simulink model "AcasXuClosedLoop_all_2017b"  (Simulink 9.0)
settings: Solver=VariableStepAuto  StartTime=0.0  StopTime=20  RelTol=1e-3
libraries: neural x14040, simulink x2
(part 12 of 534)

== system AcasXuClosedLoop_all_2017b/networks/Networks_m_2/AcasXu_4_2/Layer 3 ==
  "a{2} " : Inport  PortDimensions=50
      mask Display: e = 0.06;c1 = [120 230 180]/255;c2 = ([120 230 180]+0)/(256+128);c3 = ([120 230 180]+128)/(256+128);patch([0 1 1 0],[0 0 1 1],c1);patch([0 1 1 1-e 1-e 0+e],[0 0 1 1-e 0+e 0+e],c2);patch([0 0 1 1-e 0+e 0+e],[0 1 1 1-e 1-e 0+e],c3);plot([0 1 1 0 0],[0 0 1 1 0]);
  Delays 1 : Subsystem
      mask Display: e = 0.06;c1 = [120 150 230]/255;c2 = ([120 150 230]+0)/383;c3 = ([120 150 230]+128)/383;patch([0 1 1 0],[0 0 1 1],c1);patch([0 1 1 1-e 1-e 0+e],[0 0 1 1-e 0+e 0+e],c2);patch([0 0 1 1-e 0+e 0+e],[0 1 1 1-e 1-e 0+e],c3);plot([0 1 1 0 0],[0 0 1 1 0]);disp('0');
  "LW{3,2}" : Subsystem
      mask Display: e = 0.06;c1 = [120 150 230]/255;c2 = ([120 150 230]+0)/383;c3 = ([120 150 230]+128)/383;patch([0 1 1 0],[0 0 1 1],c1);patch([0 1 1 1-e 1-e 0+e],[0 0 1 1-e 0+e 0+e],c2);patch([0 0 1 1-e 0+e 0+e],[0 1 1 1-e 1-e 0+e],c3);plot([0 1 1 0 0],[0 0 1 1 0]);disp('W');
  "b{3}" : Constant  Value=[-1.2093100000000001070787902790470980107784271240234375;-0.3852510000000000101039177025086246430873870849609375;-1.1825900000000000300559577226522378623485565185546875;0.373638999999999998902211473250645212829113006591796875;-1.0167500000000000426325641456060111522674560546875;-0.17659700000000000397193389289896003901958465576171875;-0.289461000000000023835156071072560735046863555908203125;-1.035530000000000061533000916824676096439361572265625;0.197462999999999999634070491083548404276371002197265625;-0.62217000000000000081712414612411521375179290771484375;-1.1047800000000000952837808654294349253177642822265625;1.0904000000000000358824081558850593864917755126953125;-0.0993316999999999949988449543525348417460918426513671875;0.144187000000000009602985073797754012048244476318359375;-0.01483650000000000072908346027134030009619891643524169921875;0.123421000000000002927436071331612765789031982421875;-1.0655699999999999061373046060907654464244842529296875;-0.013062600000000000599609251139554544351994991302490234375;-0.5942600000000000104449782156734727323055267333984375;-0.4652439999999999908908421275555156171321868896484375;-0.395979000000000025405455517102382145822048187255859375;-0.0127361000000000001985966946449480019509792327880859375;-1.1045100000000001028155338644864968955516815185546875;-0.1830179999999999862492217062026611529290676116943359375;0.90672699999999994968646888082730583846569061279296875;-0.57416999999999995818456000051810406148433685302734375;-0.6274889999999999634638925272156484425067901611328125;0.69388600000000000278532752417959272861480712890625;-0.285387000000000001787014980436651967465877532958984375;1.4988099999999999756283841634285636246204376220703125;0.04194029999999999980264675514263217337429523468017578125;-0.39841300000000001713118535917601548135280609130859375;-0.486005999999999993566035527692292816936969757080078125;-1.3225100000000000743938244340824894607067108154296875;0.375159000000000020236257114447653293609619140625;-0.42299100000000000587618842473602853715419769287109375;0.38622800000000001574562702444382011890411376953125;-1.039639999999999897539737503393553197383880615234375;-1.2919899999999999717914533903240226209163665771484375;0.93974400000000002375344365646014921367168426513671875;-0.371230000000000004423128530106623657047748565673828125;-0.34603200000000000624567064733128063380718231201171875;-0.36628899999999997572075471907737664878368377685546875;-1.4668200000000000127187149701057933270931243896484375;0.79338900000000001089262013920233584940433502197265625;-0.47510799999999997478283830787404440343379974365234375;0.552489000000000007872813512221910059452056884765625;2.261649999999999938182781988871283829212188720703125;1.162819999999999964757080306299030780792236328125;0.258203000000000015834444866413832642138004302978515625]
      mask Display: e = 0.06;c1 = [120 150 230]/255;c2 = ([120 150 230]+0)/383;c3 = ([120 150 230]+128)/383;patch([0 1 1 0],[0 0 1 1],c1);patch([0 1 1 1-e 1-e 0+e],[0 0 1 1-e 0+e 0+e],c2);patch([0 0 1 1-e 0+e 0+e],[0 1 1 1-e 1-e 0+e],c3);plot([0 1 1 0 0],[0 0 1 1 0]);disp('b');
  netsum : Sum  Inputs=2  SaturateOnIntegerOverflow=on  InputSameDT=on  OutDataTypeStr=Inherit: Same as first input
      mask Display: e = 0.06;c1 = [120 150 230]/255;c2 = ([120 150 230]+0)/383;c3 = ([120 150 230]+128)/383;patch([0 1 1 0],[0 0 1 1],c1);patch([0 1 1 1-e 1-e 0+e],[0 0 1 1-e 0+e 0+e],c2);patch([0 0 1 1-e 0+e 0+e],[0 1 1 1-e 1-e 0+e],c3);plot([0 1 1 0 0],[0 0 1 1 0]);disp('+');
  poslin : neural/Transfer Functions/poslin
      settings: SourceProductName=Deep Learning Toolbox  SourceProductBaseCode=NN
  "a{3}" : Outport  InitialOutput=[0;0;0;0;0;0;0;0;0;0;0;0;0;0;0;0;0;0;0;0;0;0;0;0;0;0;0;0;0;0;0;0;0;0;0;0;0;0;0;0;0;0;0;0;0;0;0;0;0;0]
      mask Display: e = 0.06;c1 = [120 230 180]/255;c2 = ([120 230 180]+0)/(256+128);c3 = ([120 230 180]+128)/(256+128);patch([0 1 1 0],[0 0 1 1],c1);patch([0 1 1 1-e 1-e 0+e],[0 0 1 1-e 0+e 0+e],c2);patch([0 0 1 1-e 0+e 0+e],[0 1 1 1-e 1-e 0+e],c3);plot([0 1 1 0 0],[0 0 1 1 0]);
  wires:
  poslin/1 -> "a{3}"/1
  netsum/1 -> poslin/1
  "b{3}"/1 -> netsum/2
  "LW{3,2}"/1("lz{3,2}") -> netsum/1
  Delays 1/1("pd{3,2}") -> "LW{3,2}"/1("ad{3,2}")
  "a{2} "/1 -> Delays 1/1("p{3}")

== system AcasXuClosedLoop_all_2017b/networks/Networks_m_2/AcasXu_4_2/Layer 4 ==
  "a{3} " : Inport  PortDimensions=50
      mask Display: e = 0.06;c1 = [120 230 180]/255;c2 = ([120 230 180]+0)/(256+128);c3 = ([120 230 180]+128)/(256+128);patch([0 1 1 0],[0 0 1 1],c1);patch([0 1 1 1-e 1-e 0+e],[0 0 1 1-e 0+e 0+e],c2);patch([0 0 1 1-e 0+e 0+e],[0 1 1 1-e 1-e 0+e],c3);plot([0 1 1 0 0],[0 0 1 1 0]);
  Delays 1 : Subsystem
      mask Display: e = 0.06;c1 = [120 150 230]/255;c2 = ([120 150 230]+0)/383;c3 = ([120 150 230]+128)/383;patch([0 1 1 0],[0 0 1 1],c1);patch([0 1 1 1-e 1-e 0+e],[0 0 1 1-e 0+e 0+e],c2);patch([0 0 1 1-e 0+e 0+e],[0 1 1 1-e 1-e 0+e],c3);plot([0 1 1 0 0],[0 0 1 1 0]);disp('0');
  "LW{4,3}" : Subsystem
      mask Display: e = 0.06;c1 = [120 150 230]/255;c2 = ([120 150 230]+0)/383;c3 = ([120 150 230]+128)/383;patch([0 1 1 0],[0 0 1 1],c1);patch([0 1 1 1-e 1-e 0+e],[0 0 1 1-e 0+e 0+e],c2);patch([0 0 1 1-e 0+e 0+e],[0 1 1 1-e 1-e 0+e],c3);plot([0 1 1 0 0],[0 0 1 1 0]);disp('W');
  "b{4}" : Constant  Value=[-0.48296499999999997765343096034484915435314178466796875;0.75015200000000004099121042600017972290515899658203125;-1.2147600000000000619593265582807362079620361328125;0.591026999999999969048758430290035903453826904296875;0.382948000000000010611955758577096275985240936279296875;0.6420369999999999688355956095620058476924896240234375;0.696061999999999958532725941040553152561187744140625;1.0299799999999998956212721168412826955318450927734375;-0.66000800000000003908695589416311122477054595947265625;-0.6410000000000000142108547152020037174224853515625;-0.1299849999999999894395585897655109874904155731201171875;0.30073600000000000331823457599966786801815032958984375;0.55291199999999995906563299286062829196453094482421875;0.26601200000000002621192152219009585678577423095703125;0.653343000000000007077005648170597851276397705078125;0.307491000000000014313883411887218244373798370361328125;-0.1523029999999999939408468208057456649839878082275390625;0.6957649999999999668176542400033213198184967041015625;0.11466300000000000103295150211124564521014690399169921875;0.54934000000000005048406137575511820614337921142578125;0.0273350000000000016575629757653587148524820804595947265625;-1.7362500000000000710542735760100185871124267578125;0.40176800000000001400479732183157466351985931396484375;-1.5132499999999999840127884453977458178997039794921875;-0.9088220000000000187156956599210388958454132080078125;-0.68895300000000003759481614906690083444118499755859375;-1.105890000000000039648284655413590371608734130859375;0.454156999999999977379872007077210582792758941650390625;1.407739999999999991331378623726777732372283935546875;0.42157400000000000428457269663340412080287933349609375;-0.441346999999999989316989967846893705427646636962890625;0.128890000000000004565237077258643694221973419189453125;-0.349038000000000014910739309925702400505542755126953125;-0.159445000000000003392841563254478387534618377685546875;0.152144000000000001460165321987005881965160369873046875;0.97558000000000000273558953267638571560382843017578125;0.01782229999999999925375249176795477978885173797607421875;0.5652470000000000549533751836861483752727508544921875;-0.01870410000000000128039800983970053493976593017578125;-0.370516999999999985249132805620320141315460205078125;-0.2447870000000000045847770024920464493334293365478515625;0.80906900000000003814193405560217797756195068359375;-0.448537000000000019017676322619081474840641021728515625;0.07267600000000000448618919790533254854381084442138671875;0.1906960000000000043929304638368193991482257843017578125;-0.0632713000000000025391244662387180142104625701904296875;0.6713750000000000550670620214077644050121307373046875;-2.451470000000000037942982089589349925518035888671875;0.0585482999999999975671016727574169635772705078125;-0.60775900000000004919087359667173586785793304443359375]
      mask Display: e = 0.06;c1 = [120 150 230]/255;c2 = ([120 150 230]+0)/383;c3 = ([120 150 230]+128)/383;patch([0 1 1 0],[0 0 1 1],c1);patch([0 1 1 1-e 1-e 0+e],[0 0 1 1-e 0+e 0+e],c2);patch([0 0 1 1-e 0+e 0+e],[0 1 1 1-e 1-e 0+e],c3);plot([0 1 1 0 0],[0 0 1 1 0]);disp('b');
  netsum : Sum  Inputs=2  SaturateOnIntegerOverflow=on  InputSameDT=on  OutDataTypeStr=Inherit: Same as first input
      mask Display: e = 0.06;c1 = [120 150 230]/255;c2 = ([120 150 230]+0)/383;c3 = ([120 150 230]+128)/383;patch([0 1 1 0],[0 0 1 1],c1);patch([0 1 1 1-e 1-e 0+e],[0 0 1 1-e 0+e 0+e],c2);patch([0 0 1 1-e 0+e 0+e],[0 1 1 1-e 1-e 0+e],c3);plot([0 1 1 0 0],[0 0 1 1 0]);disp('+');
  poslin : neural/Transfer Functions/poslin
      settings: SourceProductName=Deep Learning Toolbox  SourceProductBaseCode=NN
  "a{4}" : Outport  InitialOutput=[0;0;0;0;0;0;0;0;0;0;0;0;0;0;0;0;0;0;0;0;0;0;0;0;0;0;0;0;0;0;0;0;0;0;0;0;0;0;0;0;0;0;0;0;0;0;0;0;0;0]
      mask Display: e = 0.06;c1 = [120 230 180]/255;c2 = ([120 230 180]+0)/(256+128);c3 = ([120 230 180]+128)/(256+128);patch([0 1 1 0],[0 0 1 1],c1);patch([0 1 1 1-e 1-e 0+e],[0 0 1 1-e 0+e 0+e],c2);patch([0 0 1 1-e 0+e 0+e],[0 1 1 1-e 1-e 0+e],c3);plot([0 1 1 0 0],[0 0 1 1 0]);
  wires:
  poslin/1 -> "a{4}"/1
  netsum/1 -> poslin/1
  "b{4}"/1 -> netsum/2
  "LW{4,3}"/1("lz{4,3}") -> netsum/1
  Delays 1/1("pd{4,3}") -> "LW{4,3}"/1("ad{4,3}")
  "a{3} "/1 -> Delays 1/1("p{4}")

== system AcasXuClosedLoop_all_2017b/networks/Networks_m_2/AcasXu_4_2/Layer 5 ==
  "a{4} " : Inport  PortDimensions=50
      mask Display: e = 0.06;c1 = [120 230 180]/255;c2 = ([120 230 180]+0)/(256+128);c3 = ([120 230 180]+128)/(256+128);patch([0 1 1 0],[0 0 1 1],c1);patch([0 1 1 1-e 1-e 0+e],[0 0 1 1-e 0+e 0+e],c2);patch([0 0 1 1-e 0+e 0+e],[0 1 1 1-e 1-e 0+e],c3);plot([0 1 1 0 0],[0 0 1 1 0]);
  Delays 1 : Subsystem
      mask Display: e = 0.06;c1 = [120 150 230]/255;c2 = ([120 150 230]+0)/383;c3 = ([120 150 230]+128)/383;patch([0 1 1 0],[0 0 1 1],c1);patch([0 1 1 1-e 1-e 0+e],[0 0 1 1-e 0+e 0+e],c2);patch([0 0 1 1-e 0+e 0+e],[0 1 1 1-e 1-e 0+e],c3);plot([0 1 1 0 0],[0 0 1 1 0]);disp('0');
  "LW{5,4}" : Subsystem
      mask Display: e = 0.06;c1 = [120 150 230]/255;c2 = ([120 150 230]+0)/383;c3 = ([120 150 230]+128)/383;patch([0 1 1 0],[0 0 1 1],c1);patch([0 1 1 1-e 1-e 0+e],[0 0 1 1-e 0+e 0+e],c2);patch([0 0 1 1-e 0+e 0+e],[0 1 1 1-e 1-e 0+e],c3);plot([0 1 1 0 0],[0 0 1 1 0]);disp('W');
  "b{5}" : Constant  Value=[1.667030000000000011795009413617663085460662841796875;-0.16405300000000000437694325228221714496612548828125;-0.036280899999999997873612045395930181257426738739013671875;-1.4923200000000000908784159037168137729167938232421875;-0.01533149999999999964217511916331204702146351337432861328125;0.89175999999999999712230192017159424722194671630859375;-1.570840000000000014068746168049983680248260498046875;-1.1088999999999999968025576890795491635799407958984375;0.75545799999999996288124748389236629009246826171875;0.43142000000000002568611989772762171924114227294921875;0.48276499999999999968025576890795491635799407958984375;1.13823000000000007503331289626657962799072265625;-1.658139999999999947277729006600566208362579345703125;-0.03238660000000000149800172266623121686279773712158203125;0.79739400000000004720135393654345534741878509521484375;-0.489690000000000014157564010019996203482151031494140625;-0.54653600000000002179234570576227270066738128662109375;0.61249500000000001165290086646564304828643798828125;0.65238899999999999668176542400033213198184967041015625;-1.121070000000000010942358130705542862415313720703125;0.64722699999999999675992512493394315242767333984375;0.5347910000000000163566937771975062787532806396484375;0.5378220000000000222684093387215398252010345458984375;-0.30079800000000000981259518084698356688022613525390625;-0.7375469999999999526352212342317216098308563232421875;-0.05225459999999999827213770231537637300789356231689453125;-0.01783490000000000075264239285388612188398838043212890625;1.2560599999999999543121020906255580484867095947265625;0.10386099999999999499156189131099381484091281890869140625;-0.34737099999999998534150336126913316547870635986328125;0.484240999999999977010389784481958486139774322509765625;0.2878249999999999975131004248396493494510650634765625;-0.6927529999999999521520521739148534834384918212890625;0.764796000000000031349145501735620200634002685546875;-0.033029799999999998050270733074285089969635009765625;-1.329590000000000049595882956054992973804473876953125;0.326909999999999978381737264498951844871044158935546875;0.1570979999999999876525436093288590200245380401611328125;0.4335620000000000029416469260468147695064544677734375;-0.10705199999999999438404785223610815592110157012939453125;-0.395469000000000014960477301428909413516521453857421875;-0.5508070000000000465689709017169661819934844970703125;0.363134000000000012331469179116538725793361663818359375;0.1310679999999999900683889109131996519863605499267578125;0.48874099999999998100719267313252203166484832763671875;-0.72436500000000003662847802843316458165645599365234375;0.53715699999999999558752961092977784574031829833984375;1.0689699999999999757704927105805836617946624755859375;-0.86060800000000003961986294598318636417388916015625;-0.032118599999999997152144715073518455028533935546875]
      mask Display: e = 0.06;c1 = [120 150 230]/255;c2 = ([120 150 230]+0)/383;c3 = ([120 150 230]+128)/383;patch([0 1 1 0],[0 0 1 1],c1);patch([0 1 1 1-e 1-e 0+e],[0 0 1 1-e 0+e 0+e],c2);patch([0 0 1 1-e 0+e 0+e],[0 1 1 1-e 1-e 0+e],c3);plot([0 1 1 0 0],[0 0 1 1 0]);disp('b');
  netsum : Sum  Inputs=2  SaturateOnIntegerOverflow=on  InputSameDT=on  OutDataTypeStr=Inherit: Same as first input
      mask Display: e = 0.06;c1 = [120 150 230]/255;c2 = ([120 150 230]+0)/383;c3 = ([120 150 230]+128)/383;patch([0 1 1 0],[0 0 1 1],c1);patch([0 1 1 1-e 1-e 0+e],[0 0 1 1-e 0+e 0+e],c2);patch([0 0 1 1-e 0+e 0+e],[0 1 1 1-e 1-e 0+e],c3);plot([0 1 1 0 0],[0 0 1 1 0]);disp('+');
  poslin : neural/Transfer Functions/poslin
      settings: SourceProductName=Deep Learning Toolbox  SourceProductBaseCode=NN
  "a{5}" : Outport  InitialOutput=[0;0;0;0;0;0;0;0;0;0;0;0;0;0;0;0;0;0;0;0;0;0;0;0;0;0;0;0;0;0;0;0;0;0;0;0;0;0;0;0;0;0;0;0;0;0;0;0;0;0]
      mask Display: e = 0.06;c1 = [120 230 180]/255;c2 = ([120 230 180]+0)/(256+128);c3 = ([120 230 180]+128)/(256+128);patch([0 1 1 0],[0 0 1 1],c1);patch([0 1 1 1-e 1-e 0+e],[0 0 1 1-e 0+e 0+e],c2);patch([0 0 1 1-e 0+e 0+e],[0 1 1 1-e 1-e 0+e],c3);plot([0 1 1 0 0],[0 0 1 1 0]);
  wires:
  poslin/1 -> "a{5}"/1
  netsum/1 -> poslin/1
  "b{5}"/1 -> netsum/2
  "LW{5,4}"/1("lz{5,4}") -> netsum/1
  Delays 1/1("pd{5,4}") -> "LW{5,4}"/1("ad{5,4}")
  "a{4} "/1 -> Delays 1/1("p{5}")

== system AcasXuClosedLoop_all_2017b/networks/Networks_m_2/AcasXu_4_2/Layer 6 ==
  "a{5} " : Inport  PortDimensions=50
      mask Display: e = 0.06;c1 = [120 230 180]/255;c2 = ([120 230 180]+0)/(256+128);c3 = ([120 230 180]+128)/(256+128);patch([0 1 1 0],[0 0 1 1],c1);patch([0 1 1 1-e 1-e 0+e],[0 0 1 1-e 0+e 0+e],c2);patch([0 0 1 1-e 0+e 0+e],[0 1 1 1-e 1-e 0+e],c3);plot([0 1 1 0 0],[0 0 1 1 0]);
  Delays 1 : Subsystem
      mask Display: e = 0.06;c1 = [120 150 230]/255;c2 = ([120 150 230]+0)/383;c3 = ([120 150 230]+128)/383;patch([0 1 1 0],[0 0 1 1],c1);patch([0 1 1 1-e 1-e 0+e],[0 0 1 1-e 0+e 0+e],c2);patch([0 0 1 1-e 0+e 0+e],[0 1 1 1-e 1-e 0+e],c3);plot([0 1 1 0 0],[0 0 1 1 0]);disp('0');
  "LW{6,5}" : Subsystem
      mask Display: e = 0.06;c1 = [120 150 230]/255;c2 = ([120 150 230]+0)/383;c3 = ([120 150 230]+128)/383;patch([0 1 1 0],[0 0 1 1],c1);patch([0 1 1 1-e 1-e 0+e],[0 0 1 1-e 0+e 0+e],c2);patch([0 0 1 1-e 0+e 0+e],[0 1 1 1-e 1-e 0+e],c3);plot([0 1 1 0 0],[0 0 1 1 0]);disp('W');
  "b{6}" : Constant  Value=[0.271396000000000026108892825504881329834461212158203125;-0.14563599999999998768629438927746377885341644287109375;0.009515980000000000271764832859844318591058254241943359375;-0.112346000000000001417532757841399870812892913818359375;-0.6355239999999999778168557895696721971035003662109375;-0.045955000000000002791100683907643542625010013580322265625;0.2134729999999999960902385964800487272441387176513671875;-1.18484999999999995878852132591418921947479248046875;0.2363860000000000127773347458060015924274921417236328125;-0.153476000000000001310951347477384842932224273681640625;-0.64991600000000004921929530610213987529277801513671875;-0.359387999999999985245580091941519640386104583740234375;-0.63418200000000002347633198951371014118194580078125;-0.206660000000000010356160373703460209071636199951171875;0.056250399999999999012256779451490729115903377532958984375;0.047399400000000001254019110774606815539300441741943359375;0.4504230000000000178062009581481106579303741455078125;-0.58878099999999999880628820392303168773651123046875;0.08031670000000000475548489475841051898896694183349609375;-2.12455000000000016058265828178264200687408447265625;-0.633866999999999958248508846736513078212738037109375;0.248182999999999986950882657765760086476802825927734375;-0.10464700000000000390532051142145064659416675567626953125;-1.4727799999999999780442294650129042565822601318359375;0.14918799999999998728839045725180767476558685302734375;0.0757379999999999997672972540385671891272068023681640625;0.471032999999999979490183932284708134829998016357421875;-0.69371499999999997054800360274384729564189910888671875;-0.32937899999999997735500301132560707628726959228515625;-1.407859999999999889297441768576391041278839111328125;0.340706000000000008842704346534446813166141510009765625;-0.0423916000000000015024426147647318430244922637939453125;-0.0020437699999999998749788954199857471394352614879608154296875;-0.06183249999999999857447363638129900209605693817138671875;0.07975949999999999706634667973048635758459568023681640625;0.2066989999999999938484762651569326408207416534423828125;-0.3627059999999999728714783486793749034404754638671875;-0.0870270999999999961271868187395739369094371795654296875;-0.2309420000000000083861806388085824437439441680908203125;-0.2197610000000000118891563261058763600885868072509765625;-0.79645799999999999929656269159750081598758697509765625;0.1211170000000000024353852268177433870732784271240234375;-0.07685419999999999751683077420238987542688846588134765625;-0.06845890000000000308322256614701473154127597808837890625;0.135371999999999992336796594827319495379924774169921875;0.2409030000000000060200733287274488247931003570556640625;0.042481499999999998429256464760328526608645915985107421875;0.275341000000000002412292587905540131032466888427734375;0.145892999999999994908961298278882168233394622802734375;-0.029134000000000000174527059471074608154594898223876953125]
      mask Display: e = 0.06;c1 = [120 150 230]/255;c2 = ([120 150 230]+0)/383;c3 = ([120 150 230]+128)/383;patch([0 1 1 0],[0 0 1 1],c1);patch([0 1 1 1-e 1-e 0+e],[0 0 1 1-e 0+e 0+e],c2);patch([0 0 1 1-e 0+e 0+e],[0 1 1 1-e 1-e 0+e],c3);plot([0 1 1 0 0],[0 0 1 1 0]);disp('b');
  netsum : Sum  Inputs=2  SaturateOnIntegerOverflow=on  InputSameDT=on  OutDataTypeStr=Inherit: Same as first input
      mask Display: e = 0.06;c1 = [120 150 230]/255;c2 = ([120 150 230]+0)/383;c3 = ([120 150 230]+128)/383;patch([0 1 1 0],[0 0 1 1],c1);patch([0 1 1 1-e 1-e 0+e],[0 0 1 1-e 0+e 0+e],c2);patch([0 0 1 1-e 0+e 0+e],[0 1 1 1-e 1-e 0+e],c3);plot([0 1 1 0 0],[0 0 1 1 0]);disp('+');
  poslin : neural/Transfer Functions/poslin
      settings: SourceProductName=Deep Learning Toolbox  SourceProductBaseCode=NN
  "a{6}" : Outport  InitialOutput=[0;0;0;0;0;0;0;0;0;0;0;0;0;0;0;0;0;0;0;0;0;0;0;0;0;0;0;0;0;0;0;0;0;0;0;0;0;0;0;0;0;0;0;0;0;0;0;0;0;0]
      mask Display: e = 0.06;c1 = [120 230 180]/255;c2 = ([120 230 180]+0)/(256+128);c3 = ([120 230 180]+128)/(256+128);patch([0 1 1 0],[0 0 1 1],c1);patch([0 1 1 1-e 1-e 0+e],[0 0 1 1-e 0+e 0+e],c2);patch([0 0 1 1-e 0+e 0+e],[0 1 1 1-e 1-e 0+e],c3);plot([0 1 1 0 0],[0 0 1 1 0]);
  wires:
  poslin/1 -> "a{6}"/1
  netsum/1 -> poslin/1
  "b{6}"/1 -> netsum/2
  "LW{6,5}"/1("lz{6,5}") -> netsum/1
  Delays 1/1("pd{6,5}") -> "LW{6,5}"/1("ad{6,5}")
  "a{5} "/1 -> Delays 1/1("p{6}")

== system AcasXuClosedLoop_all_2017b/networks/Networks_m_2/AcasXu_4_2/Layer 7 ==
  "a{6} " : Inport  PortDimensions=50
      mask Display: e = 0.06;c1 = [120 230 180]/255;c2 = ([120 230 180]+0)/(256+128);c3 = ([120 230 180]+128)/(256+128);patch([0 1 1 0],[0 0 1 1],c1);patch([0 1 1 1-e 1-e 0+e],[0 0 1 1-e 0+e 0+e],c2);patch([0 0 1 1-e 0+e 0+e],[0 1 1 1-e 1-e 0+e],c3);plot([0 1 1 0 0],[0 0 1 1 0]);
  Delays 1 : Subsystem
      mask Display: e = 0.06;c1 = [120 150 230]/255;c2 = ([120 150 230]+0)/383;c3 = ([120 150 230]+128)/383;patch([0 1 1 0],[0 0 1 1],c1);patch([0 1 1 1-e 1-e 0+e],[0 0 1 1-e 0+e 0+e],c2);patch([0 0 1 1-e 0+e 0+e],[0 1 1 1-e 1-e 0+e],c3);plot([0 1 1 0 0],[0 0 1 1 0]);disp('0');
  "LW{7,6}" : Subsystem
      mask Display: e = 0.06;c1 = [120 150 230]/255;c2 = ([120 150 230]+0)/383;c3 = ([120 150 230]+128)/383;patch([0 1 1 0],[0 0 1 1],c1);patch([0 1 1 1-e 1-e 0+e],[0 0 1 1-e 0+e 0+e],c2);patch([0 0 1 1-e 0+e 0+e],[0 1 1 1-e 1-e 0+e],c3);plot([0 1 1 0 0],[0 0 1 1 0]);disp('W');
  "b{7}" : Constant  Value=[0.01468369999999999918338655646721235825680196285247802734375;-0.00470128000000000038915981548370837117545306682586669921875;0.035427699999999999469491029913115198723971843719482421875;-0.000654970999999999960346219740614515103516168892383575439453125;0.03616529999999999744897394293730030767619609832763671875]
      mask Display: e = 0.06;c1 = [120 150 230]/255;c2 = ([120 150 230]+0)/383;c3 = ([120 150 230]+128)/383;patch([0 1 1 0],[0 0 1 1],c1);patch([0 1 1 1-e 1-e 0+e],[0 0 1 1-e 0+e 0+e],c2);patch([0 0 1 1-e 0+e 0+e],[0 1 1 1-e 1-e 0+e],c3);plot([0 1 1 0 0],[0 0 1 1 0]);disp('b');
  netsum : Sum  Inputs=2  SaturateOnIntegerOverflow=on  InputSameDT=on  OutDataTypeStr=Inherit: Same as first input
      mask Display: e = 0.06;c1 = [120 150 230]/255;c2 = ([120 150 230]+0)/383;c3 = ([120 150 230]+128)/383;patch([0 1 1 0],[0 0 1 1],c1);patch([0 1 1 1-e 1-e 0+e],[0 0 1 1-e 0+e 0+e],c2);patch([0 0 1 1-e 0+e 0+e],[0 1 1 1-e 1-e 0+e],c3);plot([0 1 1 0 0],[0 0 1 1 0]);disp('+');
  purelin : neural/Transfer Functions/purelin
      settings: SourceProductName=Deep Learning Toolbox
  "a{7}" : Outport  InitialOutput=[0;0;0;0;0]
      mask Display: e = 0.06;c1 = [120 230 180]/255;c2 = ([120 230 180]+0)/(256+128);c3 = ([120 230 180]+128)/(256+128);patch([0 1 1 0],[0 0 1 1],c1);patch([0 1 1 1-e 1-e 0+e],[0 0 1 1-e 0+e 0+e],c2);patch([0 0 1 1-e 0+e 0+e],[0 1 1 1-e 1-e 0+e],c3);plot([0 1 1 0 0],[0 0 1 1 0]);
  wires:
  purelin/1 -> "a{7}"/1
  netsum/1 -> purelin/1
  "b{7}"/1 -> netsum/2
  "LW{7,6}"/1("lz{7,6}") -> netsum/1
  Delays 1/1("pd{7,6}") -> "LW{7,6}"/1("ad{7,6}")
  "a{6} "/1 -> Delays 1/1("p{7}")

== system AcasXuClosedLoop_all_2017b/networks/Networks_m_2/AcasXu_4_2/Process Input 1 ==
  x : Inport  PortDimensions=5
      mask Display: e = 0.06;c1 = [120 230 180]/255;c2 = ([120 230 180]+0)/(256+128);c3 = ([120 230 180]+128)/(256+128);patch([0 1 1 0],[0 0 1 1],c1);patch([0 1 1 1-e 1-e 0+e],[0 0 1 1-e 0+e 0+e],c2);patch([0 0 1 1-e 0+e 0+e],[0 1 1 1-e 1-e 0+e],c3);plot([0 1 1 0 0],[0 0 1 1 0]);
  p : Outport  PortDimensions=5
      mask Display: e = 0.06;c1 = [120 230 180]/255;c2 = ([120 230 180]+0)/(256+128);c3 = ([120 230 180]+128)/(256+128);patch([0 1 1 0],[0 0 1 1],c1);patch([0 1 1 1-e 1-e 0+e],[0 0 1 1-e 0+e 0+e],c2);patch([0 0 1 1-e 0+e 0+e],[0 1 1 1-e 1-e 0+e],c3);plot([0 1 1 0 0],[0 0 1 1 0]);
  wires:
  x/1 -> p/1

== system AcasXuClosedLoop_all_2017b/networks/Networks_m_2/AcasXu_4_2/Process Output 1 ==
  a : Inport  PortDimensions=5
      mask Display: e = 0.06;c1 = [120 230 180]/255;c2 = ([120 230 180]+0)/(256+128);c3 = ([120 230 180]+128)/(256+128);patch([0 1 1 0],[0 0 1 1],c1);patch([0 1 1 1-e 1-e 0+e],[0 0 1 1-e 0+e 0+e],c2);patch([0 0 1 1-e 0+e 0+e],[0 1 1 1-e 1-e 0+e],c3);plot([0 1 1 0 0],[0 0 1 1 0]);
  y : Outport  PortDimensions=5
      mask Display: e = 0.06;c1 = [120 230 180]/255;c2 = ([120 230 180]+0)/(256+128);c3 = ([120 230 180]+128)/(256+128);patch([0 1 1 0],[0 0 1 1],c1);patch([0 1 1 1-e 1-e 0+e],[0 0 1 1-e 0+e 0+e],c2);patch([0 0 1 1-e 0+e 0+e],[0 1 1 1-e 1-e 0+e],c3);plot([0 1 1 0 0],[0 0 1 1 0]);
  wires:
  a/1 -> y/1

== system AcasXuClosedLoop_all_2017b/networks/Networks_m_2/AcasXu_5_2/Layer 1 ==
  "p{1}" : Inport  PortDimensions=5
      mask Display: e = 0.06;c1 = [120 230 180]/255;c2 = ([120 230 180]+0)/(256+128);c3 = ([120 230 180]+128)/(256+128);patch([0 1 1 0],[0 0 1 1],c1);patch([0 1 1 1-e 1-e 0+e],[0 0 1 1-e 0+e 0+e],c2);patch([0 0 1 1-e 0+e 0+e],[0 1 1 1-e 1-e 0+e],c3);plot([0 1 1 0 0],[0 0 1 1 0]);
  Delays 1 : Subsystem
      mask Display: e = 0.06;c1 = [120 150 230]/255;c2 = ([120 150 230]+0)/383;c3 = ([120 150 230]+128)/383;patch([0 1 1 0],[0 0 1 1],c1);patch([0 1 1 1-e 1-e 0+e],[0 0 1 1-e 0+e 0+e],c2);patch([0 0 1 1-e 0+e 0+e],[0 1 1 1-e 1-e 0+e],c3);plot([0 1 1 0 0],[0 0 1 1 0]);disp('0');
  "IW{1,1}" : Subsystem
      mask Display: e = 0.06;c1 = [120 150 230]/255;c2 = ([120 150 230]+0)/383;c3 = ([120 150 230]+128)/383;patch([0 1 1 0],[0 0 1 1],c1);patch([0 1 1 1-e 1-e 0+e],[0 0 1 1-e 0+e 0+e],c2);patch([0 0 1 1-e 0+e 0+e],[0 1 1 1-e 1-e 0+e],c3);plot([0 1 1 0 0],[0 0 1 1 0]);disp('W');
  "b{1}" : Constant  Value=[-0.5225699999999999789679350215010344982147216796875;0.08098919999999999730366795347435981966555118560791015625;-0.388828000000000006952660669412580318748950958251953125;-0.423870000000000024531487952117458917200565338134765625;0.363358000000000014306777984529617242515087127685546875;0.113070000000000003836930773104541003704071044921875;-0.007512839999999999796020944131669239141047000885009765625;-0.44695099999999998718891447424539364874362945556640625;-0.024655400000000000926281273905260604806244373321533203125;0.00349412999999999980549336697777107474394142627716064453125;0.1640500000000000013766765505351941101253032684326171875;0.151363999999999998546940105370595119893550872802734375;-0.7931340000000000056701310313655994832515716552734375;-0.257234000000000018193446749137365259230136871337890625;-0.044330799999999996596766749235030147247016429901123046875;-0.1867379999999999873327993782368139363825321197509765625;0.047071899999999999852917653697659261524677276611328125;-0.11033800000000000551825252159687806852161884307861328125;-0.526672000000000029018565328442491590976715087890625;-0.294516999999999973258724139668629504740238189697265625;-0.01289189999999999954038987226567769539542496204376220703125;0.13947100000000001163158458439284004271030426025390625;-0.7452140000000000430446789323468692600727081298828125;-0.3850379999999999913740111878723837435245513916015625;-0.1279039999999999899227276500823791138827800750732421875;-0.1974829999999999918802728871014551259577274322509765625;0.2259859999999999924380489346731337718665599822998046875;-0.41572799999999998643573917433968745172023773193359375;0.0689332000000000000294875235340441577136516571044921875;-0.31816100000000002712141622396302409470081329345703125;0.54194399999999998129851519479416310787200927734375;-0.10822600000000000275424127949008834548294544219970703125;-0.427329999999999987636556397774256765842437744140625;-0.465955999999999981309173335830564610660076141357421875;-0.5985270000000000312212478092988021671772003173828125;-0.0249557000000000007988720795992776402272284030914306640625;-0.492057000000000022144064359963522292673587799072265625;-0.76232200000000005513101086762617342174053192138671875;0.060068000000000003335554055183820310048758983612060546875;-0.7915039999999999853486087886267341673374176025390625;0.25127500000000002611244553918368183076381683349609375;0.1326779999999999903881331420052447356283664703369140625;0.62141900000000005466205266202450729906558990478515625;-0.56192600000000003657163460957235656678676605224609375;0.093970100000000000850519654704839922487735748291015625;-0.0257846000000000012686296457786738756112754344940185546875;0.008458040000000000002700062395888380706310272216796875;-0.2342750000000000110134124042815528810024261474609375;-0.1987899999999999944844120136622223071753978729248046875;-0.0026113299999999998145250312830967232002876698970794677734375]
      mask Display: e = 0.06;c1 = [120 150 230]/255;c2 = ([120 150 230]+0)/383;c3 = ([120 150 230]+128)/383;patch([0 1 1 0],[0 0 1 1],c1);patch([0 1 1 1-e 1-e 0+e],[0 0 1 1-e 0+e 0+e],c2);patch([0 0 1 1-e 0+e 0+e],[0 1 1 1-e 1-e 0+e],c3);plot([0 1 1 0 0],[0 0 1 1 0]);disp('b');
  netsum : Sum  Inputs=2  SaturateOnIntegerOverflow=on  InputSameDT=on  OutDataTypeStr=Inherit: Same as first input
      mask Display: e = 0.06;c1 = [120 150 230]/255;c2 = ([120 150 230]+0)/383;c3 = ([120 150 230]+128)/383;patch([0 1 1 0],[0 0 1 1],c1);patch([0 1 1 1-e 1-e 0+e],[0 0 1 1-e 0+e 0+e],c2);patch([0 0 1 1-e 0+e 0+e],[0 1 1 1-e 1-e 0+e],c3);plot([0 1 1 0 0],[0 0 1 1 0]);disp('+');
  poslin : neural/Transfer Functions/poslin
      settings: SourceProductName=Deep Learning Toolbox  SourceProductBaseCode=NN
  "a{1}" : Outport  InitialOutput=[0;0;0;0;0;0;0;0;0;0;0;0;0;0;0;0;0;0;0;0;0;0;0;0;0;0;0;0;0;0;0;0;0;0;0;0;0;0;0;0;0;0;0;0;0;0;0;0;0;0]
      mask Display: e = 0.06;c1 = [120 230 180]/255;c2 = ([120 230 180]+0)/(256+128);c3 = ([120 230 180]+128)/(256+128);patch([0 1 1 0],[0 0 1 1],c1);patch([0 1 1 1-e 1-e 0+e],[0 0 1 1-e 0+e 0+e],c2);patch([0 0 1 1-e 0+e 0+e],[0 1 1 1-e 1-e 0+e],c3);plot([0 1 1 0 0],[0 0 1 1 0]);
  wires:
  poslin/1 -> "a{1}"/1
  netsum/1 -> poslin/1
  "b{1}"/1 -> netsum/2
  "IW{1,1}"/1("iz{1,1}") -> netsum/1
  Delays 1/1("pd{1,1}") -> "IW{1,1}"/1("pd{1,1}")
  "p{1}"/1 -> Delays 1/1("p{1}")

== system AcasXuClosedLoop_all_2017b/networks/Networks_m_2/AcasXu_5_2/Layer 2 ==
  "a{1} " : Inport  PortDimensions=50
      mask Display: e = 0.06;c1 = [120 230 180]/255;c2 = ([120 230 180]+0)/(256+128);c3 = ([120 230 180]+128)/(256+128);patch([0 1 1 0],[0 0 1 1],c1);patch([0 1 1 1-e 1-e 0+e],[0 0 1 1-e 0+e 0+e],c2);patch([0 0 1 1-e 0+e 0+e],[0 1 1 1-e 1-e 0+e],c3);plot([0 1 1 0 0],[0 0 1 1 0]);
  Delays 1 : Subsystem
      mask Display: e = 0.06;c1 = [120 150 230]/255;c2 = ([120 150 230]+0)/383;c3 = ([120 150 230]+128)/383;patch([0 1 1 0],[0 0 1 1],c1);patch([0 1 1 1-e 1-e 0+e],[0 0 1 1-e 0+e 0+e],c2);patch([0 0 1 1-e 0+e 0+e],[0 1 1 1-e 1-e 0+e],c3);plot([0 1 1 0 0],[0 0 1 1 0]);disp('0');
  "LW{2,1}" : Subsystem
      mask Display: e = 0.06;c1 = [120 150 230]/255;c2 = ([120 150 230]+0)/383;c3 = ([120 150 230]+128)/383;patch([0 1 1 0],[0 0 1 1],c1);patch([0 1 1 1-e 1-e 0+e],[0 0 1 1-e 0+e 0+e],c2);patch([0 0 1 1-e 0+e 0+e],[0 1 1 1-e 1-e 0+e],c3);plot([0 1 1 0 0],[0 0 1 1 0]);disp('W');
  "b{2}" : Constant  Value=[-0.89502199999999998425437297555617988109588623046875;-0.018699500000000000843325409505268908105790615081787109375;0.2032090000000000007407408020299044437706470489501953125;0.58568600000000003991118546764482744038105010986328125;-0.30132199999999997874766677341540344059467315673828125;0.2235290000000000054658499948345706798136234283447265625;0.42994399999999999284483465089579112827777862548828125;-0.10915999999999999314770349201353383250534534454345703125;0.1981320000000000025597302055757609196007251739501953125;0.251230000000000008864020628607249818742275238037109375;0.405830999999999997296384890432818792760372161865234375;-0.88307100000000005035616368331830017268657684326171875;-0.858354999999999979110043568653054535388946533203125;-0.0498197999999999974196640550871961750090122222900390625;0.56830999999999998184563310132944025099277496337890625;-0.0176161999999999985266896374014322645962238311767578125;0.63321799999999994756905152826220728456974029541015625;-1.20436999999999994059862729045562446117401123046875;-0.4421260000000000189857018995098769664764404296875;-0.126738999999999990553334328069468028843402862548828125;-0.77897600000000000175504055732744745910167694091796875;0.1111209999999999975539566321458551101386547088623046875;-0.08144220000000000625473006721222191117703914642333984375;-0.37489899999999998225774788807029835879802703857421875;-0.66500000000000003552713678800500929355621337890625;-0.00811400999999999962553065557813170016743242740631103515625;-0.2423639999999999961044494511952507309615612030029296875;0.377323000000000019493739955578348599374294281005859375;-0.01467830000000000002791100683907643542625010013580322265625;0.01024680000000000035242919693700969219207763671875;-0.4687580000000000080007112046587280929088592529296875;-0.0150302999999999999103383885312723577953875064849853515625;-0.0088615099999999995261656948741801897995173931121826171875;-0.062033600000000001239985891743344836868345737457275390625;0.62612199999999995636557059697224758565425872802734375;-0.0793092999999999992422061723118531517684459686279296875;-0.0152160999999999997644994920165117946453392505645751953125;0.85023099999999995901589500135742127895355224609375;0.51935600000000003984013119406881742179393768310546875;0.123840000000000005631051180898793973028659820556640625;0.412412999999999974054532003719941712915897369384765625;-0.0257520000000000005846434447676074341870844364166259765625;-1.13956999999999997186250766390003263950347900390625;-0.412250999999999978573583803154178895056247711181640625;0.5354769999999999807727135703316889703273773193359375;-0.016002099999999998269917256266126059927046298980712890625;-0.05118169999999999664197503079776652157306671142578125;0.60169700000000003736744247362366877496242523193359375;0.551595999999999975216269376687705516815185546875;-0.0310881999999999998618438468156455201096832752227783203125]
      mask Display: e = 0.06;c1 = [120 150 230]/255;c2 = ([120 150 230]+0)/383;c3 = ([120 150 230]+128)/383;patch([0 1 1 0],[0 0 1 1],c1);patch([0 1 1 1-e 1-e 0+e],[0 0 1 1-e 0+e 0+e],c2);patch([0 0 1 1-e 0+e 0+e],[0 1 1 1-e 1-e 0+e],c3);plot([0 1 1 0 0],[0 0 1 1 0]);disp('b');
  netsum : Sum  Inputs=2  SaturateOnIntegerOverflow=on  InputSameDT=on  OutDataTypeStr=Inherit: Same as first input
      mask Display: e = 0.06;c1 = [120 150 230]/255;c2 = ([120 150 230]+0)/383;c3 = ([120 150 230]+128)/383;patch([0 1 1 0],[0 0 1 1],c1);patch([0 1 1 1-e 1-e 0+e],[0 0 1 1-e 0+e 0+e],c2);patch([0 0 1 1-e 0+e 0+e],[0 1 1 1-e 1-e 0+e],c3);plot([0 1 1 0 0],[0 0 1 1 0]);disp('+');
  poslin : neural/Transfer Functions/poslin
      settings: SourceProductName=Deep Learning Toolbox  SourceProductBaseCode=NN
  "a{2}" : Outport  InitialOutput=[0;0;0;0;0;0;0;0;0;0;0;0;0;0;0;0;0;0;0;0;0;0;0;0;0;0;0;0;0;0;0;0;0;0;0;0;0;0;0;0;0;0;0;0;0;0;0;0;0;0]
      mask Display: e = 0.06;c1 = [120 230 180]/255;c2 = ([120 230 180]+0)/(256+128);c3 = ([120 230 180]+128)/(256+128);patch([0 1 1 0],[0 0 1 1],c1);patch([0 1 1 1-e 1-e 0+e],[0 0 1 1-e 0+e 0+e],c2);patch([0 0 1 1-e 0+e 0+e],[0 1 1 1-e 1-e 0+e],c3);plot([0 1 1 0 0],[0 0 1 1 0]);
  wires:
  poslin/1 -> "a{2}"/1
  netsum/1 -> poslin/1
  "b{2}"/1 -> netsum/2
  "LW{2,1}"/1("lz{2,1}") -> netsum/1
  Delays 1/1("pd{2,1}") -> "LW{2,1}"/1("ad{2,1}")
  "a{1} "/1 -> Delays 1/1("p{2}")

== system AcasXuClosedLoop_all_2017b/networks/Networks_m_2/AcasXu_5_2/Layer 3 ==
  "a{2} " : Inport  PortDimensions=50
      mask Display: e = 0.06;c1 = [120 230 180]/255;c2 = ([120 230 180]+0)/(256+128);c3 = ([120 230 180]+128)/(256+128);patch([0 1 1 0],[0 0 1 1],c1);patch([0 1 1 1-e 1-e 0+e],[0 0 1 1-e 0+e 0+e],c2);patch([0 0 1 1-e 0+e 0+e],[0 1 1 1-e 1-e 0+e],c3);plot([0 1 1 0 0],[0 0 1 1 0]);
  Delays 1 : Subsystem
      mask Display: e = 0.06;c1 = [120 150 230]/255;c2 = ([120 150 230]+0)/383;c3 = ([120 150 230]+128)/383;patch([0 1 1 0],[0 0 1 1],c1);patch([0 1 1 1-e 1-e 0+e],[0 0 1 1-e 0+e 0+e],c2);patch([0 0 1 1-e 0+e 0+e],[0 1 1 1-e 1-e 0+e],c3);plot([0 1 1 0 0],[0 0 1 1 0]);disp('0');
  "LW{3,2}" : Subsystem
      mask Display: e = 0.06;c1 = [120 150 230]/255;c2 = ([120 150 230]+0)/383;c3 = ([120 150 230]+128)/383;patch([0 1 1 0],[0 0 1 1],c1);patch([0 1 1 1-e 1-e 0+e],[0 0 1 1-e 0+e 0+e],c2);patch([0 0 1 1-e 0+e 0+e],[0 1 1 1-e 1-e 0+e],c3);plot([0 1 1 0 0],[0 0 1 1 0]);disp('W');
  "b{3}" : Constant  Value=[-0.64185800000000003961986294598318636417388916015625;-0.6596290000000000208757455766317434608936309814453125;-1.545269999999999921413973424932919442653656005859375;0.8008699999999999707966935602598823606967926025390625;0.2160849999999999992983390484369010664522647857666015625;-0.1297439999999999982183140900815487839281558990478515625;-0.137012999999999995903721128343022428452968597412109375;-0.364929000000000003378630708539276383817195892333984375;-0.267378999999999977799092221175669692456722259521484375;0.225785999999999986709298127607326023280620574951171875;-1.3678600000000000758149099056026898324489593505859375;-0.0257474000000000001475708444331758073531091213226318359375;0.60395699999999996610000607688562013208866119384765625;-0.332050000000000011812772982011665590107440948486328125;-0.5530209999999999848085963094490580260753631591796875;-0.850009000000000014551915228366851806640625;0.2122739999999999904733982702964567579329013824462890625;0.35152200000000000112976294985855929553508758544921875;-1.0495900000000000229505303650512360036373138427734375;0.379643000000000008231637593780760653316974639892578125;-0.0181869999999999983064657982367862132377922534942626953125;-2.90833999999999992525090419803746044635772705078125;-0.182800999999999991274535204865969717502593994140625;-0.089695999999999997953636921010911464691162109375;-0.45067299999999999027266994744422845542430877685546875;0.50790699999999999736388645033002831041812896728515625;0.383670000000000011031175972675555385649204254150390625;0.057920199999999998075050910983918583951890468597412109375;-0.0952994000000000063277383333115722052752971649169921875;-0.599960999999999966547648000414483249187469482421875;-0.18777399999999999646860260327230207622051239013671875;-0.094381199999999998428990011234418489038944244384765625;0.185574999999999989963583857388584874570369720458984375;-1.2059800000000000519406739840633235871791839599609375;-0.8804380000000000539017719347612001001834869384765625;-1.0353600000000000580513415116001851856708526611328125;-0.28331600000000001227107304657693020999431610107421875;0.470447000000000004060751734868972562253475189208984375;0.170752999999999988123278171769925393164157867431640625;0.021825799999999999367883418699420872144401073455810546875;-0.10570000000000000228705943072782247327268123626708984375;0.25540200000000001789857151379692368209362030029296875;-0.87033499999999996976640659340773709118366241455078125;-1.2368099999999999649702431270270608365535736083984375;-0.034925900000000002776356922140621463768184185028076171875;-0.8665169999999999816964191268198192119598388671875;-0.6342689999999999717061882620328105986118316650390625;-0.210096000000000004970246436641900800168514251708984375;-0.465515999999999985359266929663135670125484466552734375;0.25755600000000000715516534910420887172222137451171875]
      mask Display: e = 0.06;c1 = [120 150 230]/255;c2 = ([120 150 230]+0)/383;c3 = ([120 150 230]+128)/383;patch([0 1 1 0],[0 0 1 1],c1);patch([0 1 1 1-e 1-e 0+e],[0 0 1 1-e 0+e 0+e],c2);patch([0 0 1 1-e 0+e 0+e],[0 1 1 1-e 1-e 0+e],c3);plot([0 1 1 0 0],[0 0 1 1 0]);disp('b');
  netsum : Sum  Inputs=2  SaturateOnIntegerOverflow=on  InputSameDT=on  OutDataTypeStr=Inherit: Same as first input
      mask Display: e = 0.06;c1 = [120 150 230]/255;c2 = ([120 150 230]+0)/383;c3 = ([120 150 230]+128)/383;patch([0 1 1 0],[0 0 1 1],c1);patch([0 1 1 1-e 1-e 0+e],[0 0 1 1-e 0+e 0+e],c2);patch([0 0 1 1-e 0+e 0+e],[0 1 1 1-e 1-e 0+e],c3);plot([0 1 1 0 0],[0 0 1 1 0]);disp('+');
  poslin : neural/Transfer Functions/poslin
      settings: SourceProductName=Deep Learning Toolbox  SourceProductBaseCode=NN
  "a{3}" : Outport  InitialOutput=[0;0;0;0;0;0;0;0;0;0;0;0;0;0;0;0;0;0;0;0;0;0;0;0;0;0;0;0;0;0;0;0;0;0;0;0;0;0;0;0;0;0;0;0;0;0;0;0;0;0]
      mask Display: e = 0.06;c1 = [120 230 180]/255;c2 = ([120 230 180]+0)/(256+128);c3 = ([120 230 180]+128)/(256+128);patch([0 1 1 0],[0 0 1 1],c1);patch([0 1 1 1-e 1-e 0+e],[0 0 1 1-e 0+e 0+e],c2);patch([0 0 1 1-e 0+e 0+e],[0 1 1 1-e 1-e 0+e],c3);plot([0 1 1 0 0],[0 0 1 1 0]);
  wires:
  poslin/1 -> "a{3}"/1
  netsum/1 -> poslin/1
  "b{3}"/1 -> netsum/2
  "LW{3,2}"/1("lz{3,2}") -> netsum/1
  Delays 1/1("pd{3,2}") -> "LW{3,2}"/1("ad{3,2}")
  "a{2} "/1 -> Delays 1/1("p{3}")

== system AcasXuClosedLoop_all_2017b/networks/Networks_m_2/AcasXu_5_2/Layer 4 ==
  "a{3} " : Inport  PortDimensions=50
      mask Display: e = 0.06;c1 = [120 230 180]/255;c2 = ([120 230 180]+0)/(256+128);c3 = ([120 230 180]+128)/(256+128);patch([0 1 1 0],[0 0 1 1],c1);patch([0 1 1 1-e 1-e 0+e],[0 0 1 1-e 0+e 0+e],c2);patch([0 0 1 1-e 0+e 0+e],[0 1 1 1-e 1-e 0+e],c3);plot([0 1 1 0 0],[0 0 1 1 0]);
  Delays 1 : Subsystem
      mask Display: e = 0.06;c1 = [120 150 230]/255;c2 = ([120 150 230]+0)/383;c3 = ([120 150 230]+128)/383;patch([0 1 1 0],[0 0 1 1],c1);patch([0 1 1 1-e 1-e 0+e],[0 0 1 1-e 0+e 0+e],c2);patch([0 0 1 1-e 0+e 0+e],[0 1 1 1-e 1-e 0+e],c3);plot([0 1 1 0 0],[0 0 1 1 0]);disp('0');
  "LW{4,3}" : Subsystem
      mask Display: e = 0.06;c1 = [120 150 230]/255;c2 = ([120 150 230]+0)/383;c3 = ([120 150 230]+128)/383;patch([0 1 1 0],[0 0 1 1],c1);patch([0 1 1 1-e 1-e 0+e],[0 0 1 1-e 0+e 0+e],c2);patch([0 0 1 1-e 0+e 0+e],[0 1 1 1-e 1-e 0+e],c3);plot([0 1 1 0 0],[0 0 1 1 0]);disp('W');
  "b{4}" : Constant  Value=[-0.0760487999999999997324806599863222800195217132568359375;-0.58008199999999998652810972998850047588348388671875;0.498491000000000017422507880837656557559967041015625;-1.1341900000000000314770431941724382340908050537109375;-0.1335860000000000102904351706456509418785572052001953125;-0.67121200000000003083044930463074706494808197021484375;0.01339360000000000029685143232427435577847063541412353515625;-0.30365100000000000424194013248779810965061187744140625;-0.322552000000000005375255796025157906115055084228515625;-0.1674710000000000087450047203674330376088619232177734375;1.025279999999999969162445268011651933193206787109375;-0.005051360000000000398390209710441922652535140514373779296875;-1.0328299999999999148059259823639877140522003173828125;-0.1916150000000000075406347832540632225573062896728515625;1.1007800000000000917310671866289339959621429443359375;-0.53687399999999996236255128678749315440654754638671875;1.689810000000000034248159863636828958988189697265625;-1.24662000000000006139089236967265605926513671875;0.69325099999999995059596358260023407638072967529296875;0.83030700000000001725908305161283351480960845947265625;0.3140950000000000130739863379858434200286865234375;-0.03511899999999999744115797284393920563161373138427734375;-0.39132000000000000117239551400416530668735504150390625;-0.168339999999999989643839626296539790928363800048828125;0.01315020000000000077733375292154960334300994873046875;1.321660000000000056985527407960034906864166259765625;0.62143899999999996364152821115567348897457122802734375;-0.0048020600000000003559907924000071943737566471099853515625;1.7843999999999999861444166526780463755130767822265625;0.07537750000000000005773159728050814010202884674072265625;-1.1433500000000000884625706021324731409549713134765625;-0.88756199999999996208543961984105408191680908203125;-1.5487800000000000455457893622224219143390655517578125;0.91866999999999998660626943092211149632930755615234375;-0.00822531999999999950679008264842195785604417324066162109375;-0.93284599999999995301180888418457470834255218505859375;-0.267123999999999972576603113338933326303958892822265625;-2.0828199999999998937028067302890121936798095703125;0.5875550000000000494537744089029729366302490234375;0.17389799999999999702282593716518022119998931884765625;-1.2074499999999999122479721336276270449161529541015625;-0.1955639999999999878443901479840860702097415924072265625;-0.547973999999999961119101499207317829132080078125;-1.427869999999999972573050399660132825374603271484375;-0.81063099999999999045741105874185450375080108642578125;0.0286893000000000009119816013480885885655879974365234375;0.491777999999999992919441638150601647794246673583984375;1.3428599999999999425881469505839049816131591796875;0.37703100000000000502353714182390831410884857177734375;-0.0241742999999999992610799637304808129556477069854736328125]
      mask Display: e = 0.06;c1 = [120 150 230]/255;c2 = ([120 150 230]+0)/383;c3 = ([120 150 230]+128)/383;patch([0 1 1 0],[0 0 1 1],c1);patch([0 1 1 1-e 1-e 0+e],[0 0 1 1-e 0+e 0+e],c2);patch([0 0 1 1-e 0+e 0+e],[0 1 1 1-e 1-e 0+e],c3);plot([0 1 1 0 0],[0 0 1 1 0]);disp('b');
  netsum : Sum  Inputs=2  SaturateOnIntegerOverflow=on  InputSameDT=on  OutDataTypeStr=Inherit: Same as first input
      mask Display: e = 0.06;c1 = [120 150 230]/255;c2 = ([120 150 230]+0)/383;c3 = ([120 150 230]+128)/383;patch([0 1 1 0],[0 0 1 1],c1);patch([0 1 1 1-e 1-e 0+e],[0 0 1 1-e 0+e 0+e],c2);patch([0 0 1 1-e 0+e 0+e],[0 1 1 1-e 1-e 0+e],c3);plot([0 1 1 0 0],[0 0 1 1 0]);disp('+');
  poslin : neural/Transfer Functions/poslin
      settings: SourceProductName=Deep Learning Toolbox  SourceProductBaseCode=NN
  "a{4}" : Outport  InitialOutput=[0;0;0;0;0;0;0;0;0;0;0;0;0;0;0;0;0;0;0;0;0;0;0;0;0;0;0;0;0;0;0;0;0;0;0;0;0;0;0;0;0;0;0;0;0;0;0;0;0;0]
      mask Display: e = 0.06;c1 = [120 230 180]/255;c2 = ([120 230 180]+0)/(256+128);c3 = ([120 230 180]+128)/(256+128);patch([0 1 1 0],[0 0 1 1],c1);patch([0 1 1 1-e 1-e 0+e],[0 0 1 1-e 0+e 0+e],c2);patch([0 0 1 1-e 0+e 0+e],[0 1 1 1-e 1-e 0+e],c3);plot([0 1 1 0 0],[0 0 1 1 0]);
  wires:
  poslin/1 -> "a{4}"/1
  netsum/1 -> poslin/1
  "b{4}"/1 -> netsum/2
  "LW{4,3}"/1("lz{4,3}") -> netsum/1
  Delays 1/1("pd{4,3}") -> "LW{4,3}"/1("ad{4,3}")
  "a{3} "/1 -> Delays 1/1("p{4}")
